simulink model "CCMDcMotor"  (R2022b)
settings: Solver=FixedStepDiscrete  StartTime=0.0  StopTime=10.0  FixedStep=0.01  RelTol=1e-3
model workspace (MAT data in the model file):
  NvMBlockDcMtrCalData : Simulink.Signal (value not decoded)
  NvMBlockDcMtrCalDataInit : Simulink.Parameter (value not decoded)
symbols that are objects in the model workspace (value not decoded): NvMBlockDcMtrCalDataInit
undefined symbols (not defined in the file or in its folder): DTCState, NvMBlockId, P_Std_ReturnType, Tle91ChlType, Tle91CmdType
(part 3 of 3)

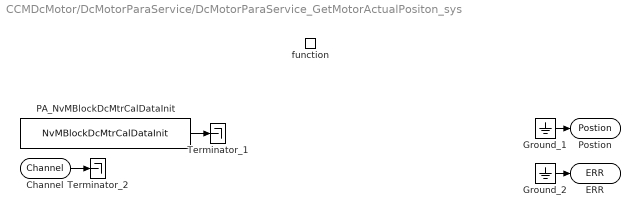
== system CCMDcMotor/DcMotorParaService/DcMotorParaService_GetMotorActualPositon_sys ==
  function : TriggerPort  TriggerType=function-call  IsSimulinkFunction=on  FunctionName=DcMotorParaService_GetMotorActualPositon  VariantControl=(inherit)
  Ground_1 : Ground
  Ground_2 : Ground
  PA_NvMBlockDcMtrCalDataInit : Constant  Value=NvMBlockDcMtrCalDataInit
  Terminator_1 : Terminator
  Terminator_2 : Terminator
  Channel : ArgIn  OutDataTypeStr=Enum: DcChlType  ArgumentName=Channel
  Postion : ArgOut  OutDataTypeStr=UInt16  ArgumentName=Postion
  ERR : ArgOut  Port=2  OutDataTypeStr=Std_ReturnType  ArgumentName=ERR
  wires:
  PA_NvMBlockDcMtrCalDataInit/1 -> Terminator_1/1
  Channel/1 -> Terminator_2/1
  Ground_1/1 -> Postion/1
  Ground_2/1 -> ERR/1

== system CCMDcMotor/DcMotorParaService/DcMotorParaService_GetMotorCailLearnSts_sys ==
  function : TriggerPort  TriggerType=function-call  IsSimulinkFunction=on  FunctionName=DcMotorParaService_GetMotorCailLearnSts  VariantControl=(inherit)
  Ground_1 : Ground
  Ground_2 : Ground
  PA_NvMBlockDcMtrCalDataInit : Constant  Value=NvMBlockDcMtrCalDataInit
  Terminator_1 : Terminator
  Status : ArgOut  OutDataTypeStr=UInt8  ArgumentName=Status
  ERR : ArgOut  Port=2  OutDataTypeStr=Std_ReturnType  ArgumentName=ERR
  wires:
  PA_NvMBlockDcMtrCalDataInit/1 -> Terminator_1/1
  Ground_1/1 -> Status/1
  Ground_2/1 -> ERR/1

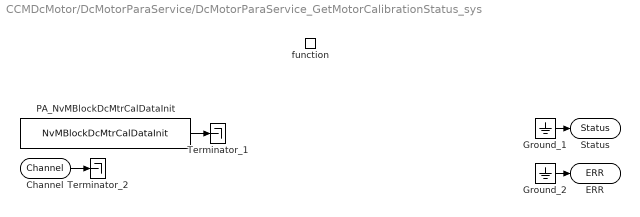
== system CCMDcMotor/DcMotorParaService/DcMotorParaService_GetMotorCalibrationStatus_sys ==
  function : TriggerPort  TriggerType=function-call  IsSimulinkFunction=on  FunctionName=DcMotorParaService_GetMotorCalibrationStatus  VariantControl=(inherit)
  Ground_1 : Ground
  Ground_2 : Ground
  PA_NvMBlockDcMtrCalDataInit : Constant  Value=NvMBlockDcMtrCalDataInit
  Terminator_1 : Terminator
  Terminator_2 : Terminator
  Channel : ArgIn  OutDataTypeStr=Enum: DcChlType  ArgumentName=Channel
  Status : ArgOut  OutDataTypeStr=UInt8  ArgumentName=Status
  ERR : ArgOut  Port=2  OutDataTypeStr=Std_ReturnType  ArgumentName=ERR
  wires:
  PA_NvMBlockDcMtrCalDataInit/1 -> Terminator_1/1
  Channel/1 -> Terminator_2/1
  Ground_1/1 -> Status/1
  Ground_2/1 -> ERR/1

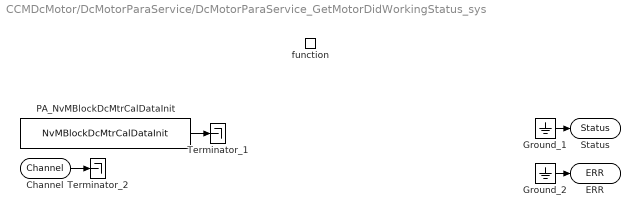
== system CCMDcMotor/DcMotorParaService/DcMotorParaService_GetMotorDidWorkingStatus_sys ==
  function : TriggerPort  TriggerType=function-call  IsSimulinkFunction=on  FunctionName=DcMotorParaService_GetMotorDidWorkingStatus  VariantControl=(inherit)
  Ground_1 : Ground
  Ground_2 : Ground
  PA_NvMBlockDcMtrCalDataInit : Constant  Value=NvMBlockDcMtrCalDataInit
  Terminator_1 : Terminator
  Terminator_2 : Terminator
  Channel : ArgIn  OutDataTypeStr=Enum: DcChlType  ArgumentName=Channel
  Status : ArgOut  OutDataTypeStr=UInt8  ArgumentName=Status
  ERR : ArgOut  Port=2  OutDataTypeStr=Std_ReturnType  ArgumentName=ERR
  wires:
  PA_NvMBlockDcMtrCalDataInit/1 -> Terminator_1/1
  Channel/1 -> Terminator_2/1
  Ground_1/1 -> Status/1
  Ground_2/1 -> ERR/1

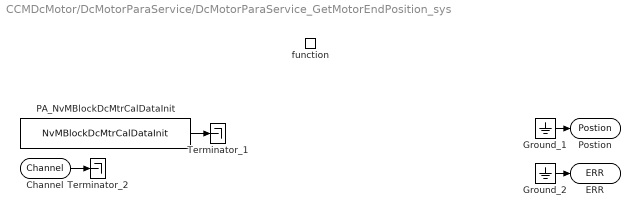
== system CCMDcMotor/DcMotorParaService/DcMotorParaService_GetMotorEndPosition_sys ==
  function : TriggerPort  TriggerType=function-call  IsSimulinkFunction=on  FunctionName=DcMotorParaService_GetMotorEndPosition  VariantControl=(inherit)
  Ground_1 : Ground
  Ground_2 : Ground
  PA_NvMBlockDcMtrCalDataInit : Constant  Value=NvMBlockDcMtrCalDataInit
  Terminator_1 : Terminator
  Terminator_2 : Terminator
  Channel : ArgIn  OutDataTypeStr=Enum: DcChlType  ArgumentName=Channel
  Postion : ArgOut  OutDataTypeStr=UInt16  ArgumentName=Postion
  ERR : ArgOut  Port=2  OutDataTypeStr=Std_ReturnType  ArgumentName=ERR
  wires:
  PA_NvMBlockDcMtrCalDataInit/1 -> Terminator_1/1
  Channel/1 -> Terminator_2/1
  Ground_1/1 -> Postion/1
  Ground_2/1 -> ERR/1

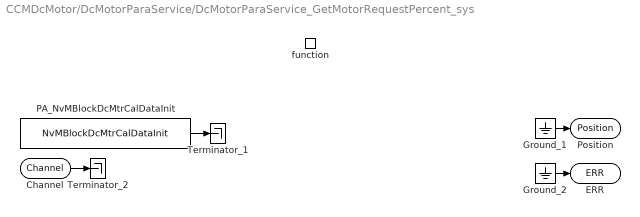
== system CCMDcMotor/DcMotorParaService/DcMotorParaService_GetMotorRequestPercent_sys ==
  function : TriggerPort  TriggerType=function-call  IsSimulinkFunction=on  FunctionName=DcMotorParaService_GetMotorRequestPercent  VariantControl=(inherit)
  Ground_1 : Ground
  Ground_2 : Ground
  PA_NvMBlockDcMtrCalDataInit : Constant  Value=NvMBlockDcMtrCalDataInit
  Terminator_1 : Terminator
  Terminator_2 : Terminator
  Channel : ArgIn  OutDataTypeStr=Enum: DcChlType  ArgumentName=Channel
  Position : ArgOut  OutDataTypeStr=UInt16  ArgumentName=Position
  ERR : ArgOut  Port=2  OutDataTypeStr=Std_ReturnType  ArgumentName=ERR
  wires:
  PA_NvMBlockDcMtrCalDataInit/1 -> Terminator_1/1
  Channel/1 -> Terminator_2/1
  Ground_1/1 -> Position/1
  Ground_2/1 -> ERR/1

== system CCMDcMotor/DcMotorParaService/DcMotorParaService_GetMotorRoutineCailSts_sys ==
  function : TriggerPort  TriggerType=function-call  IsSimulinkFunction=on  FunctionName=DcMotorParaService_GetMotorRoutineCailSts  VariantControl=(inherit)
  Ground_1 : Ground
  Ground_2 : Ground
  PA_NvMBlockDcMtrCalDataInit : Constant  Value=NvMBlockDcMtrCalDataInit
  Terminator_1 : Terminator
  Status : ArgOut  OutDataTypeStr=UInt8  ArgumentName=Status
  ERR : ArgOut  Port=2  OutDataTypeStr=Std_ReturnType  ArgumentName=ERR
  wires:
  PA_NvMBlockDcMtrCalDataInit/1 -> Terminator_1/1
  Ground_1/1 -> Status/1
  Ground_2/1 -> ERR/1

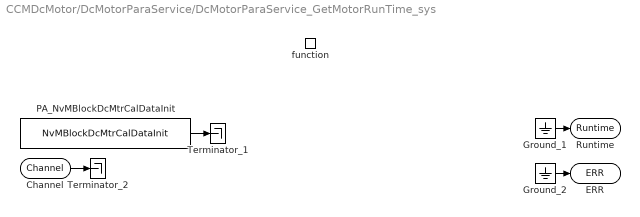
== system CCMDcMotor/DcMotorParaService/DcMotorParaService_GetMotorRunTime_sys ==
  function : TriggerPort  TriggerType=function-call  IsSimulinkFunction=on  FunctionName=DcMotorParaService_GetMotorRunTime  VariantControl=(inherit)
  Ground_1 : Ground
  Ground_2 : Ground
  PA_NvMBlockDcMtrCalDataInit : Constant  Value=NvMBlockDcMtrCalDataInit
  Terminator_1 : Terminator
  Terminator_2 : Terminator
  Channel : ArgIn  OutDataTypeStr=Enum: DcChlType  ArgumentName=Channel
  Runtime : ArgOut  OutDataTypeStr=UInt32  ArgumentName=Runtime
  ERR : ArgOut  Port=2  OutDataTypeStr=Std_ReturnType  ArgumentName=ERR
  wires:
  PA_NvMBlockDcMtrCalDataInit/1 -> Terminator_1/1
  Channel/1 -> Terminator_2/1
  Ground_1/1 -> Runtime/1
  Ground_2/1 -> ERR/1

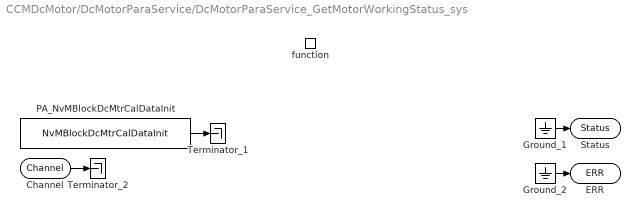
== system CCMDcMotor/DcMotorParaService/DcMotorParaService_GetMotorWorkingStatus_sys ==
  function : TriggerPort  TriggerType=function-call  IsSimulinkFunction=on  FunctionName=DcMotorParaService_GetMotorWorkingStatus  VariantControl=(inherit)
  Ground_1 : Ground
  Ground_2 : Ground
  PA_NvMBlockDcMtrCalDataInit : Constant  Value=NvMBlockDcMtrCalDataInit
  Terminator_1 : Terminator
  Terminator_2 : Terminator
  Channel : ArgIn  OutDataTypeStr=Enum: DcChlType  ArgumentName=Channel
  Status : ArgOut  OutDataTypeStr=UInt8  ArgumentName=Status
  ERR : ArgOut  Port=2  OutDataTypeStr=Std_ReturnType  ArgumentName=ERR
  wires:
  PA_NvMBlockDcMtrCalDataInit/1 -> Terminator_1/1
  Channel/1 -> Terminator_2/1
  Ground_1/1 -> Status/1
  Ground_2/1 -> ERR/1

== system CCMDcMotor/DcMotorParaService/DcMotorParaService_GetMotorZeroPosition_sys ==
  function : TriggerPort  TriggerType=function-call  IsSimulinkFunction=on  FunctionName=DcMotorParaService_GetMotorZeroPosition  VariantControl=(inherit)
  Ground_1 : Ground
  Ground_2 : Ground
  PA_NvMBlockDcMtrCalDataInit : Constant  Value=NvMBlockDcMtrCalDataInit
  Terminator_1 : Terminator
  Terminator_2 : Terminator
  Channel : ArgIn  OutDataTypeStr=Enum: DcChlType  ArgumentName=Channel
  Position : ArgOut  OutDataTypeStr=UInt16  ArgumentName=Position
  ERR : ArgOut  Port=2  OutDataTypeStr=Std_ReturnType  ArgumentName=ERR
  wires:
  PA_NvMBlockDcMtrCalDataInit/1 -> Terminator_1/1
  Channel/1 -> Terminator_2/1
  Ground_1/1 -> Position/1
  Ground_2/1 -> ERR/1

== system CCMDcMotor/DcMotorParaService/DcMotorParaService_GetRamAirErrorStatus_sys ==
  function : TriggerPort  TriggerType=function-call  IsSimulinkFunction=on  FunctionName=DcMotorParaService_GetRamAirErrorStatus  VariantControl=(inherit)
  Ground_1 : Ground
  Ground_2 : Ground
  PA_NvMBlockDcMtrCalDataInit : Constant  Value=NvMBlockDcMtrCalDataInit
  Terminator_1 : Terminator
  Status : ArgOut  OutDataTypeStr=UInt8  ArgumentName=Status
  ERR : ArgOut  Port=2  OutDataTypeStr=Std_ReturnType  ArgumentName=ERR
  wires:
  PA_NvMBlockDcMtrCalDataInit/1 -> Terminator_1/1
  Ground_1/1 -> Status/1
  Ground_2/1 -> ERR/1

== system CCMDcMotor/DcMotorParaService/DcMotorParaService_SetMotorEndPosition_sys ==
  function : TriggerPort  TriggerType=function-call  IsSimulinkFunction=on  FunctionName=DcMotorParaService_SetMotorEndPosition  VariantControl=(inherit)
  Ground_1 : Ground
  PA_NvMBlockDcMtrCalDataInit : Constant  Value=NvMBlockDcMtrCalDataInit
  Terminator_1 : Terminator
  Terminator_2 : Terminator
  Terminator_3 : Terminator
  Channel : ArgIn  OutDataTypeStr=Enum: DcChlType  ArgumentName=Channel
  Postion : ArgIn  Port=2  OutDataTypeStr=UInt16  ArgumentName=Postion
  ERR : ArgOut  OutDataTypeStr=Std_ReturnType  ArgumentName=ERR
  wires:
  PA_NvMBlockDcMtrCalDataInit/1 -> Terminator_1/1
  Channel/1 -> Terminator_2/1
  Postion/1 -> Terminator_3/1
  Ground_1/1 -> ERR/1

== system CCMDcMotor/DcMotorParaService/DcMotorParaService_SetMotorRequestPercent_sys ==
  function : TriggerPort  TriggerType=function-call  IsSimulinkFunction=on  FunctionName=DcMotorParaService_SetMotorRequestPercent  VariantControl=(inherit)
  Ground_1 : Ground
  PA_NvMBlockDcMtrCalDataInit : Constant  Value=NvMBlockDcMtrCalDataInit
  Terminator_1 : Terminator
  Terminator_2 : Terminator
  Terminator_3 : Terminator
  Channel : ArgIn  OutDataTypeStr=Enum: DcChlType  ArgumentName=Channel
  Position : ArgIn  Port=2  OutDataTypeStr=UInt16  ArgumentName=Position
  ERR : ArgOut  OutDataTypeStr=Std_ReturnType  ArgumentName=ERR
  wires:
  PA_NvMBlockDcMtrCalDataInit/1 -> Terminator_1/1
  Channel/1 -> Terminator_2/1
  Position/1 -> Terminator_3/1
  Ground_1/1 -> ERR/1

== system CCMDcMotor/DcMotorParaService/DcMotorParaService_SetMotorRoutineCailSts_sys ==
  function : TriggerPort  TriggerType=function-call  IsSimulinkFunction=on  FunctionName=DcMotorParaService_SetMotorRoutineCailSts  VariantControl=(inherit)
  Ground_1 : Ground
  PA_NvMBlockDcMtrCalDataInit : Constant  Value=NvMBlockDcMtrCalDataInit
  Terminator_1 : Terminator
  Terminator_2 : Terminator
  Status : ArgIn  OutDataTypeStr=UInt8  ArgumentName=Status
  ERR : ArgOut  OutDataTypeStr=Std_ReturnType  ArgumentName=ERR
  wires:
  PA_NvMBlockDcMtrCalDataInit/1 -> Terminator_1/1
  Status/1 -> Terminator_2/1
  Ground_1/1 -> ERR/1

== system CCMDcMotor/DcMotorParaService/DcMotorParaService_SetMotorRoutineCail_sys ==
  function : TriggerPort  TriggerType=function-call  IsSimulinkFunction=on  FunctionName=DcMotorParaService_SetMotorRoutineCail  VariantControl=(inherit)
  Ground_1 : Ground
  PA_NvMBlockDcMtrCalDataInit : Constant  Value=NvMBlockDcMtrCalDataInit
  Terminator_1 : Terminator
  ERR : ArgOut  OutDataTypeStr=Std_ReturnType  ArgumentName=ERR
  wires:
  PA_NvMBlockDcMtrCalDataInit/1 -> Terminator_1/1
  Ground_1/1 -> ERR/1

== system CCMDcMotor/DcMotorParaService/DcMotorParaService_SetMotorRunTime_sys ==
  function : TriggerPort  TriggerType=function-call  IsSimulinkFunction=on  FunctionName=DcMotorParaService_SetMotorRunTime  VariantControl=(inherit)
  Ground_1 : Ground
  PA_NvMBlockDcMtrCalDataInit : Constant  Value=NvMBlockDcMtrCalDataInit
  Terminator_1 : Terminator
  Terminator_2 : Terminator
  Terminator_3 : Terminator
  Channel : ArgIn  OutDataTypeStr=Enum: DcChlType  ArgumentName=Channel
  Runtime : ArgIn  Port=2  OutDataTypeStr=UInt32  ArgumentName=Runtime
  ERR : ArgOut  OutDataTypeStr=Std_ReturnType  ArgumentName=ERR
  wires:
  PA_NvMBlockDcMtrCalDataInit/1 -> Terminator_1/1
  Channel/1 -> Terminator_2/1
  Runtime/1 -> Terminator_3/1
  Ground_1/1 -> ERR/1

== system CCMDcMotor/DcMotorParaService/DcMotorParaService_SetMotorZeroPosition_sys ==
  function : TriggerPort  TriggerType=function-call  IsSimulinkFunction=on  FunctionName=DcMotorParaService_SetMotorZeroPosition  VariantControl=(inherit)
  Ground_1 : Ground
  PA_NvMBlockDcMtrCalDataInit : Constant  Value=NvMBlockDcMtrCalDataInit
  Terminator_1 : Terminator
  Terminator_2 : Terminator
  Terminator_3 : Terminator
  Channel : ArgIn  OutDataTypeStr=Enum: DcChlType  ArgumentName=Channel
  Position : ArgIn  Port=2  OutDataTypeStr=UInt16  ArgumentName=Position
  ERR : ArgOut  OutDataTypeStr=Std_ReturnType  ArgumentName=ERR
  wires:
  PA_NvMBlockDcMtrCalDataInit/1 -> Terminator_1/1
  Channel/1 -> Terminator_2/1
  Position/1 -> Terminator_3/1
  Ground_1/1 -> ERR/1

== system CCMDcMotor/DcMtrCaliServive/DcMtrCaliServive_SetAllCalibrate_sys ==
  function : TriggerPort  TriggerType=function-call  IsSimulinkFunction=on  FunctionName=DcMtrCaliServive_SetAllCalibrate  VariantControl=(inherit)
  Ground_1 : Ground
  PA_NvMBlockDcMtrCalDataInit : Constant  Value=NvMBlockDcMtrCalDataInit
  Terminator_1 : Terminator
  ERR : ArgOut  OutDataTypeStr=Std_ReturnType  ArgumentName=ERR
  wires:
  PA_NvMBlockDcMtrCalDataInit/1 -> Terminator_1/1
  Ground_1/1 -> ERR/1

== system CCMDcMotor/DcMtrCaliServive/DcMtrCaliServive_SetMotorCalibrate_sys ==
  function : TriggerPort  TriggerType=function-call  IsSimulinkFunction=on  FunctionName=DcMtrCaliServive_SetMotorCalibrate  VariantControl=(inherit)
  Ground_1 : Ground
  PA_NvMBlockDcMtrCalDataInit : Constant  Value=NvMBlockDcMtrCalDataInit
  Terminator_1 : Terminator
  Terminator_2 : Terminator
  Channel : ArgIn  OutDataTypeStr=UInt8  ArgumentName=Channel
  ERR : ArgOut  OutDataTypeStr=Std_ReturnType  ArgumentName=ERR
  wires:
  PA_NvMBlockDcMtrCalDataInit/1 -> Terminator_1/1
  Channel/1 -> Terminator_2/1
  Ground_1/1 -> ERR/1
